simulink model "cddDcmApp"  (R2022b) (continued)
== system cddDcmApp == (continued)
  VentnActr03ElecErr_VentnActr03ElecErr : Inport  Port=275  OutDataTypeStr=Enum: VentnActr01ElecErr  PortDimensions=1
  VentnActr03OvrTempErr_VentnActr03OvrTempErr : Inport  Port=276  OutDataTypeStr=Enum: VentnActr01OvrTempErr  PortDimensions=1
  VentnActr03VoltErr_VentnActr03VoltErr : Inport  Port=277  OutDataTypeStr=Enum: VentnActr01VoltErr  PortDimensions=1
  VentnActr04ElecErr_VentnActr04ElecErr : Inport  Port=278  OutDataTypeStr=Enum: VentnActr01ElecErr  PortDimensions=1
  VentnActr04OvrTempErr_VentnActr04OvrTempErr : Inport  Port=279  OutDataTypeStr=Enum: VentnActr01OvrTempErr  PortDimensions=1
  VentnActr04VoltErr_VentnActr04VoltErr : Inport  Port=280  OutDataTypeStr=Enum: VentnActr01VoltErr  PortDimensions=1
  VentnActr05ElecErr_VentnActr05ElecErr : Inport  Port=281  OutDataTypeStr=Enum: VentnActr01ElecErr  PortDimensions=1
  VentnActr05OvrTempErr_VentnActr05OvrTempErr : Inport  Port=282  OutDataTypeStr=Enum: VentnActr01OvrTempErr  PortDimensions=1
  VentnActr05VoltErr_VentnActr05VoltErr : Inport  Port=283  OutDataTypeStr=Enum: VentnActr01VoltErr  PortDimensions=1
  VentnActr06ElecErr_VentnActr06ElecErr : Inport  Port=284  OutDataTypeStr=Enum: VentnActr01ElecErr  PortDimensions=1
  VentnActr06OvrTempErr_VentnActr06OvrTempErr : Inport  Port=285  OutDataTypeStr=Enum: VentnActr01OvrTempErr  PortDimensions=1
  VentnActr06VoltErr_VentnActr06VoltErr : Inport  Port=286  OutDataTypeStr=Enum: VentnActr01VoltErr  PortDimensions=1
  VentnActr07ElecErr_VentnActr07ElecErr : Inport  Port=287  OutDataTypeStr=Enum: VentnActr01ElecErr  PortDimensions=1
  VentnActr07OvrTempErr_VentnActr07OvrTempErr : Inport  Port=288  OutDataTypeStr=Enum: VentnActr01OvrTempErr  PortDimensions=1
  VentnActr07VoltErr_VentnActr07VoltErr : Inport  Port=289  OutDataTypeStr=Enum: VentnActr01VoltErr  PortDimensions=1
  VentnActr08ElecErr_VentnActr08ElecErr : Inport  Port=290  OutDataTypeStr=Enum: VentnActr01ElecErr  PortDimensions=1
  VentnActr08OvrTempErr_VentnActr08OvrTempErr : Inport  Port=291  OutDataTypeStr=Enum: VentnActr01OvrTempErr  PortDimensions=1
  VentnActr08VoltErr_VentnActr08VoltErr : Inport  Port=292  OutDataTypeStr=Enum: VentnActr01VoltErr  PortDimensions=1
  VentnActr09ElecErr_VentnActr09ElecErr : Inport  Port=293  OutDataTypeStr=Enum: VentnActr01ElecErr  PortDimensions=1
  VentnActr09OvrTempErr_VentnActr09OvrTempErr : Inport  Port=294  OutDataTypeStr=Enum: VentnActr01OvrTempErr  PortDimensions=1
  VentnActr09VoltErr_VentnActr09VoltErr : Inport  Port=295  OutDataTypeStr=Enum: VentnActr01VoltErr  PortDimensions=1
  BswLifeCycle_LifeCycle : Inport  Port=296  OutDataTypeStr=Enum: CCMLifeCycleModeGroup  PortDimensions=1
  DataServices_DID_4756_DID_4756_Front_Mosfet_Blower_FB : Subsystem
  DataServices_DID_47F3_DID_47F3_AIU_Failure_Reasons_and : Subsystem
  DataServices_DID_47F4_DID_47F4_AQS_Air_Quality : Subsystem
  DataServices_DID_47F5_DID_47F5_TestRunCriteria_TRC : Subsystem
  DataServices_DID_47F6_DID_47F6_CCM_Lin1_Frame : Subsystem
  DataServices_DID_47F7_DID_47F7_CCM_Lin2_Frame : Subsystem
  DataServices_DID_47F8_DID_47F8_CCM_Lin3_Frame : Subsystem
  DataServices_DID_47F9_DID_47F9_Blower_Max_Level_Voltage : Subsystem
  DataServices_DID_4AF7_DID_4AF7_1_DC_Motor_Drive_Request_Number : Subsystem
  DataServices_DID_4AF7_DID_4AF7_2_DC_Motor_Drive_Request_Time : Subsystem
  DataServices_DID_4AF7_DID_4AF7_3_DC_Motor_Drive_Request_Direction : Subsystem
  DataServices_DID_9800_DID_9800_1_Evaporator_Temperature : Subsystem
  DataServices_DID_9800_DID_9800_2_incar_temperature : Subsystem
  DataServices_DID_9800_DID_9800_3_outside_temperature : Subsystem
  DataServices_DID_9800_DID_9800_4_Hex_sensor : Subsystem
  DataServices_DID_9801_DID_9801_1_Front_left_vent_temperature : Subsystem
  DataServices_DID_9801_DID_9801_2_Front_left_floor_temperature : Subsystem
  DataServices_DID_9801_DID_9801_3_Front_right_vent_temperature : Subsystem
  DataServices_DID_9801_DID_9801_4_Front_right_floor_temperature : Subsystem
  DataServices_DID_9802_1_Second_row_left_vent_temperatureSecond_row_vent_temperature : Subsystem
  DataServices_DID_9802_2_Second_row_left_floor_temperatureSecond_row_floor_temperature : Subsystem
  DataServices_DID_9802_DID_9802_3_Second_row_right_vent_temperature : Subsystem
  DataServices_DID_9802_DID_9802_4_Second_row_right_floor_temperature : Subsystem
  DataServices_DID_9803_DID_9803_1_Left_sun_intensity : Subsystem
  DataServices_DID_9803_DID_9803_2_Right_sun_intensity : Subsystem
  DataServices_DID_9803_DID_9803_3_engine_coolant_temperature : Subsystem
  DataServices_DID_9803_DID_9803_4_dew_temperature : Subsystem
  DataServices_DID_9804_DID_9804_1_Relative_Humidity : Subsystem
  DataServices_DID_9804_DID_9804_2_AQS_sensor : Subsystem
  DataServices_DID_9804_DID_9804_3_PM25PM25_in_sensor_concentration : Subsystem
  DataServices_DID_9804_DID_9804_4_PM25_out_sensor_concentration : Subsystem
  DataServices_DID_9805_DID_9805_1_CO2_sensor_concentration : Subsystem
  DataServices_DID_9805_DID_9805_2_Front_windscreen_temperature : Subsystem
  DataServices_DID_9805_DID_9805_3_Rear_cabin_temperature : Subsystem
  DataServices_DID_9805_DID_9805_4_Third_row_air_temperature : Subsystem
  DataServices_DID_9808_DID_9808_RHFA_Actuator_Position : Subsystem
  DataServices_DID_9809_3_actuator_positionSecond_temperature_actuator_position : Subsystem
  DataServices_DID_9809_DID_9809_1_Front_left_temperature_actuator_position : Subsystem
  DataServices_DID_9809_DID_9809_2_Front_right_temperature_actuator_position : Subsystem
  DataServices_DID_9809_DID_9809_4_Second_right_temperature_actuator_position : Subsystem
  DataServices_DID_980A_2_Front_ventfloor_actuator_positionfront_vent_actuator_position : Subsystem
  DataServices_DID_980A_DID_980A_1_Deforst_actuator_position : Subsystem
  DataServices_DID_980A_DID_980A_3_Front_floor_actuator_positionposition : Subsystem
  DataServices_DID_980A_DID_980A_4_Second_row_ventventfloor_actuator_position : Subsystem
  DataServices_DID_980B_DID_980B_10_VGA7Passenger_Right_X_actuator_positon : Subsystem
  DataServices_DID_980B_DID_980B_11_VGA8Passenger_Right_Y_actuator_positon : Subsystem
  DataServices_DID_980B_DID_980B_1_second_row_floor_actuator_position : Subsystem
  DataServices_DID_980B_DID_980B_2_Rec_actuator_position : Subsystem
  DataServices_DID_980B_DID_980B_3_Osa_actuator_position : Subsystem
  DataServices_DID_980B_DID_980B_4_VGA1Driver_Left_X_actuator_positon : Subsystem
  DataServices_DID_980B_DID_980B_5_VGA2Driver_Left_Y_actuator_positon : Subsystem
  DataServices_DID_980B_DID_980B_6_VGA3Driver_Right_X_actuator_positon : Subsystem
  DataServices_DID_980B_DID_980B_7_VGA4Driver_Right_Y_actuator_positon : Subsystem
  DataServices_DID_980B_DID_980B_8_VGA5Passenger_Left_X_actuator_positon : Subsystem
  DataServices_DID_980B_DID_980B_9_VGA6Passenger_Left_Y_actuator_positon : Subsystem
  DataServices_DID_980E_DID_980E_1_Front_Blower_pwm : Subsystem
  DataServices_DID_980E_DID_980E_2_Front_Blower_speedLIN1 : Subsystem
  DataServices_DID_980E_DID_980E_3_Incar_motorActvCmd : Subsystem
  DataServices_DID_980E_DID_980E_4_Rear_blower_speed : Subsystem
  DataServices_DID_980F_Data_Record : Subsystem
  DataServices_DID_987B_DID_987B_HBMF_Failure : Subsystem
  DataServices_DID_987C_DID_987C_HBMF_Feedback : Subsystem
  DataServices_DID_987D_DID_987D_PMSI_Failure : Subsystem
  DataServices_DID_987E_DID_987E_AFU_Failure : Subsystem
  DataServices_DID_987F_DID_987F_CCM_Low_Voltage_Power_Limited : Subsystem
  DataServices_DID_9880_DID_9880_PWM_Blower_Error_Internal_Info : Subsystem
  DataServices_DID_988E_DID_988E_Front_PWM_Blower_Feedback : Subsystem
  DataServices_DID_988F_DID_988F_Rear_Blower_Feedback : Subsystem
  DataServices_DID_9890_DID_9890_VGA1_Failure : Subsystem
  DataServices_DID_9891_DID_9891_VGA2_Failure : Subsystem
  DataServices_DID_9892_DID_9892_VGA3_Failure : Subsystem
  DataServices_DID_9893_DID_9893_VGA4_Failure : Subsystem
  DataServices_DID_9894_DID_9894_VGA5_Failure : Subsystem
  DataServices_DID_9895_DID_9895_VGA6_Failure : Subsystem
  DataServices_DID_9896_DID_9896_VGA7_Failure : Subsystem
  DataServices_DID_9897_DID_9897_VGA8_Failure : Subsystem
  DataServices_DID_9898_DID_9898_Sensor_AD : Subsystem
  DataServices_DID_989C_DID_989C_Rear_LIN_Blower_Module_VBMR_Fan_Status : Subsystem
  DataServices_DID_989D_DID_989D_Rear_LIN_Blower_Module_VBMR_Feedback_Datalog : Subsystem
  DataServices_DID_989E_DID_989E_Rear_HVAC_Flap_Actuator_RHFA_Failure_Reasons : Subsystem
  DataServices_DID_989F_DID_989F_CCM_LIN_Actuator_Frame_Status : Subsystem
  DataServices_DID_98A1_DID_98A1_98A1_DC_Motor_Feedback_Voltage : Subsystem
  DataServices_DID_98A2_DID_98A2_Front_Lpm_Blower_Feedback_Voltage_Difference : Subsystem
  DataServices_DID_98A3_DID_98A3_Advanced_Air_Cleaner_AAC_Feedback_Info : Subsystem
  DataServices_DID_98A4_DID_98A4_Oxygen_Generator_Module_OGM_Feedback_Info : Subsystem
  DataServices_DID_98A5_DID_98A5_Interior_Oxygen_Concentration_Sensor_IOSM_Module_Feedback_Info : Subsystem
  DataServices_DID_D910_DID_D910_BLW_THM_Output : Subsystem
  DataServices_DID_D922_DID_D922_1_HVAC_Front_Blower_control_level : Subsystem
  DataServices_DID_D922_DID_D922_2_HVAC_Rear_Blower_control_level : Subsystem
  DataServices_DID_D924_DID_D924_1_No_Of_Movements : Subsystem
  DataServices_DID_D924_DID_D924_2_ADC_value_Lower_Endstop_at_0 : Subsystem
  DataServices_DID_D924_DID_D924_3_ADC_value_Upper_Endstop_at_100 : Subsystem
  DataServices_DID_D925_ComplexStructure_2 : Subsystem
  DataServices_DID_D925_DID_D925_1_Requested_Position : Subsystem
  DataServices_DID_D925_DID_D925_2_Actual_Position : Subsystem
  DataServices_DID_D926_DID_D926_1_No_Of_Movements : Subsystem
  DataServices_DID_D926_DID_D926_2_ADC_value_Lower_Endstop_at_0 : Subsystem
  DataServices_DID_D926_DID_D926_3_ADC_value_Upper_Endstop_at_100 : Subsystem
  DataServices_DID_D927_ComplexStructure_2 : Subsystem
  DataServices_DID_D927_DID_D927_1_Requested_Position : Subsystem
  DataServices_DID_D927_DID_D927_2_Actual_Position : Subsystem
  DataServices_DID_D928_DID_D928_1_No_Of_Movements : Subsystem
  DataServices_DID_D928_DID_D928_2_ADC_value_Lower_Endstop_at_0 : Subsystem
  DataServices_DID_D928_DID_D928_3_ADC_value_Upper_Endstop_at_100 : Subsystem
  DataServices_DID_D929_ComplexStructure_2 : Subsystem
  DataServices_DID_D929_DID_D929_1_Requested_Position : Subsystem
  DataServices_DID_D929_DID_D929_2_Actual_Position : Subsystem
  DataServices_DID_D92A_DID_D92A_1_No_Of_Movements : Subsystem
  DataServices_DID_D92A_DID_D92A_2_ADC_value_Lower_Endstop_at_0 : Subsystem
  DataServices_DID_D92A_DID_D92A_3_ADC_value_Upper_Endstop_at_100 : Subsystem
  DataServices_DID_D92B_ComplexStructure_2 : Subsystem
  DataServices_DID_D92B_DID_D92B_1_Requested_Position : Subsystem
  DataServices_DID_D92B_DID_D92B_2_Actual_Position : Subsystem
  DataServices_DID_D92C_DID_D92C_1_No_Of_Movements : Subsystem
  DataServices_DID_D92C_DID_D92C_2_ADC_value_Lower_Endstop_at_0 : Subsystem
  DataServices_DID_D92C_DID_D92C_3_ADC_value_Upper_Endstop_at_100 : Subsystem
  DataServices_DID_D92D_ComplexStructure_2 : Subsystem
  DataServices_DID_D92D_DID_D92D_1_Requested_Position : Subsystem
  DataServices_DID_D92D_DID_D92D_2_Actual_Position : Subsystem
  DataServices_DID_D92E_DID_D92E_1_No_Of_Movements : Subsystem
  DataServices_DID_D92E_DID_D92E_2_ADC_value_Lower_Endstop_at_0 : Subsystem
  DataServices_DID_D92E_DID_D92E_3_ADC_value_Upper_Endstop_at_100 : Subsystem
  DataServices_DID_D92F_ComplexStructure_2 : Subsystem
  DataServices_DID_D92F_DID_D92F_1_Requested_Position : Subsystem
  DataServices_DID_D92F_DID_D92F_2_Actual_Position : Subsystem
  DataServices_DID_D930_DID_D930_1_No_Of_Movements : Subsystem
  DataServices_DID_D930_DID_D930_2_ADC_value_Lower_Endstop_at_0 : Subsystem
  DataServices_DID_D930_DID_D930_3_ADC_value_Upper_Endstop_at_100 : Subsystem
  DataServices_DID_D931_ComplexStructure_2 : Subsystem
  DataServices_DID_D931_DID_D931_1_Requested_Position : Subsystem
  DataServices_DID_D931_DID_D931_2_Actual_Position : Subsystem
  DataServices_DID_D933_DID_D933_1_step_Range : Subsystem
  DataServices_DID_D933_DID_D933_2_No_Of_Movements : Subsystem
  DataServices_DID_D934_ComplexStructure_2 : Subsystem
  DataServices_DID_D934_DID_D934_1_Requested_Position : Subsystem
  DataServices_DID_D934_DID_D934_2_Actual_Position : Subsystem
  DataServices_DID_D935_DID_D935_1_step_Range : Subsystem
  DataServices_DID_D935_DID_D935_2_No_Of_Movements : Subsystem
  DataServices_DID_D936_ComplexStructure_2 : Subsystem
  DataServices_DID_D936_DID_D936_1_Requested_Position : Subsystem
  DataServices_DID_D936_DID_D936_2_Actual_Position : Subsystem
  DataServices_DID_D937_DID_D937_1_step_Range : Subsystem
  DataServices_DID_D937_DID_D937_2_No_Of_Movements : Subsystem
  DataServices_DID_D938_ComplexStructure_2 : Subsystem
  DataServices_DID_D938_DID_D938_1_Requested_Position : Subsystem
  DataServices_DID_D938_DID_D938_2_Actual_Position : Subsystem
  DataServices_DID_D939_DID_D939_1_step_Range : Subsystem
  DataServices_DID_D939_DID_D939_2_No_Of_Movements : Subsystem
  DataServices_DID_D93A_ComplexStructure_2 : Subsystem
  DataServices_DID_D93A_DID_D93A_1_Requested_Position : Subsystem
  DataServices_DID_D93A_DID_D93A_2_Actual_Position : Subsystem
  DataServices_DID_D93B_DID_D93B_1_step_Range : Subsystem
  DataServices_DID_D93B_DID_D93B_2_No_Of_Movements : Subsystem
  DataServices_DID_D93C_ComplexStructure_2 : Subsystem
  DataServices_DID_D93C_DID_D93C_1_Requested_Position : Subsystem
  DataServices_DID_D93C_DID_D93C_2_Actual_Position : Subsystem
  DataServices_DID_D93D_DID_D93D_1_step_Range : Subsystem
  DataServices_DID_D93D_DID_D93D_2_No_Of_Movements : Subsystem
  DataServices_DID_D93E_ComplexStructure_2 : Subsystem
  DataServices_DID_D93E_DID_D93E_1_Requested_Position : Subsystem
  DataServices_DID_D93E_DID_D93E_2_Actual_Position : Subsystem
  DataServices_DID_D93F_DID_D93F_1_step_Range : Subsystem
  DataServices_DID_D93F_DID_D93F_2_No_Of_Movements : Subsystem
  DataServices_DID_D940_ComplexStructure_2 : Subsystem
  DataServices_DID_D940_DID_D940_1_Requested_Position : Subsystem
  DataServices_DID_D940_DID_D940_2_Actual_Position : Subsystem
  DataServices_DID_D941_DID_D941_1_step_Range : Subsystem
  DataServices_DID_D941_DID_D941_2_No_Of_Movements : Subsystem
  DataServices_DID_D942_ComplexStructure_2 : Subsystem
  DataServices_DID_D942_DID_D942_1_Requested_Position : Subsystem
  DataServices_DID_D942_DID_D942_2_Actual_Position : Subsystem
  DataServices_DID_D943_DID_D943_1_step_Range : Subsystem
  DataServices_DID_D943_DID_D943_2_No_Of_Movements : Subsystem
  DataServices_DID_D944_ComplexStructure_2 : Subsystem
  DataServices_DID_D944_DID_D944_1_Requested_Position : Subsystem
  DataServices_DID_D944_DID_D944_2_Actual_Position : Subsystem
  DataServices_DID_D945_DID_D945_1_step_Range : Subsystem
  DataServices_DID_D945_DID_D945_2_No_Of_Movements : Subsystem
  DataServices_DID_D946_ComplexStructure_2 : Subsystem
  DataServices_DID_D946_DID_D946_1_Requested_Position : Subsystem
  DataServices_DID_D946_DID_D946_2_Actual_Position : Subsystem
  DataServices_DID_D947_DID_D947_1_step_Range : Subsystem
  DataServices_DID_D947_DID_D947_2_No_Of_Movements : Subsystem
  DataServices_DID_D948_ComplexStructure_2 : Subsystem
  DataServices_DID_D948_DID_D948_1_Requested_Position : Subsystem
  DataServices_DID_D948_DID_D948_2_Actual_Position : Subsystem
  DataServices_DID_D94C_THM_SW_Version_DID_D94C_1_THM_SW_Version : Subsystem
  DataServices_DID_D94C_THM_SW_Version_DID_D94C_2_Calibration_Version : Subsystem
  DataServices_DID_D950_DID_D950_1_DC_motor_Maximum_Calibration_limit : Subsystem
  DataServices_DID_D950_DID_D950_2_DC_motor_Actual_ignition_counter : Subsystem
  DataServices_DID_D950_DID_D950_3_HFA_step_motor_Maximum_Calibration_limit : Subsystem
  DataServices_DID_D950_DID_D950_4_HFA_step_motor_Actual_ignition_counter : Subsystem
  DataServices_DID_D950_DID_D950_5_VGA_step_Maximum_Calibration_limit : Subsystem
  DataServices_DID_D950_DID_D950_6_VGA_step_Actual_ignition_counter : Subsystem
  DataServices_DID_D951_DID_D951_1_AQS_HS_Smart_Control : Subsystem
  DataServices_DID_D951_DID_D951_2_VBAT_short_circuit_detection : Subsystem
  DataServices_DID_D951_DID_D951_3_Rear_ETXV_LS_Smart_Control : Subsystem
  DataServices_DID_D951_DID_D951_4_LIN_Power_Supply : Subsystem
  DataServices_DID_D951_DID_D951_5_Rear_PTC_LS_Smart_Control : Subsystem
  DataServices_DID_D951_DID_D951_6_VGARHFA_motor_power_switch : Subsystem
  DataServices_DID_D957_DID_D957_MistRiskLvl : Subsystem
  DataServices_DID_D958_DID_D958_1_step_Range : Subsystem
  DataServices_DID_D958_DID_D958_2_No_Of_Movements : Subsystem
  DataServices_DID_D959_ComplexStructure_2 : Subsystem
  DataServices_DID_D959_DID_D959_1_Requested_Position : Subsystem
  DataServices_DID_D959_DID_D959_2_Actual_Position : Subsystem
  DataServices_DID_D95A_DID_D95A_1_step_Range : Subsystem
  DataServices_DID_D95A_DID_D95A_2_No_Of_Movements : Subsystem
  DataServices_DID_D95B_ComplexStructure_2 : Subsystem
  DataServices_DID_D95B_DID_D95B_1_Requested_Position : Subsystem
  DataServices_DID_D95B_DID_D95B_2_Actual_Position : Subsystem
  DataServices_DID_D95C_DID_D95C_1_step_Range : Subsystem
  DataServices_DID_D95C_DID_D95C_2_No_Of_Movements : Subsystem
  DataServices_DID_D95D_ComplexStructure_2 : Subsystem
  DataServices_DID_D95D_DID_D95D_1_Requested_Position : Subsystem
  DataServices_DID_D95D_DID_D95D_2_Actual_Position : Subsystem
  DataServices_DID_D95E_DID_D95E_1_step_Range : Subsystem
  DataServices_DID_D95E_DID_D95E_2_No_Of_Movements : Subsystem
  DataServices_DID_D95F_ComplexStructure_2 : Subsystem
  DataServices_DID_D95F_DID_D95F_1_Requested_Position : Subsystem
  DataServices_DID_D95F_DID_D95F_2_Actual_Position : Subsystem
  DataServices_DID_D960_DID_D960_1_step_Range : Subsystem
  DataServices_DID_D960_DID_D960_2_No_Of_Movements : Subsystem
  DataServices_DID_D961_ComplexStructure_2 : Subsystem
  DataServices_DID_D961_DID_D961_1_Requested_Position : Subsystem
  DataServices_DID_D961_DID_D961_2_Actual_Position : Subsystem
  DataServices_DID_D962_DID_D962_1_step_Range : Subsystem
  DataServices_DID_D962_DID_D962_2_No_Of_Movements : Subsystem
  DataServices_DID_D963_ComplexStructure_2 : Subsystem
  DataServices_DID_D963_DID_D963_1_Requested_Position : Subsystem
  DataServices_DID_D963_DID_D963_2_Actual_Position : Subsystem
  DataServices_DID_D964_DID_D964_1_step_Range : Subsystem
  DataServices_DID_D964_DID_D964_2_No_Of_Movements : Subsystem
  DataServices_DID_D965_ComplexStructure_2 : Subsystem
  DataServices_DID_D965_DID_D965_1_Requested_Position : Subsystem
  DataServices_DID_D965_DID_D965_2_Actual_Position : Subsystem
  DataServices_DID_D966_DID_D966_1_step_Range : Subsystem
  DataServices_DID_D966_DID_D966_2_No_Of_Movements : Subsystem
  DataServices_DID_D967_ComplexStructure_2 : Subsystem
  DataServices_DID_D967_DID_D967_1_Requested_Position : Subsystem
  DataServices_DID_D967_DID_D967_2_Actual_Position : Subsystem
  DataServices_DID_D968_DID_D968_1_step_Range : Subsystem
  DataServices_DID_D968_DID_D968_2_No_Of_Movements : Subsystem
  DataServices_DID_D969_ComplexStructure_2 : Subsystem
  DataServices_DID_D969_DID_D969_1_Requested_Position : Subsystem
  DataServices_DID_D969_DID_D969_2_Actual_Position : Subsystem
  DataServices_DID_D970_Stepper_Motor_12_Mode_Panel_First_Left_Flap_DID_D970_1_step_Range : Subsystem
  DataServices_DID_D970_Stepper_Motor_12_Mode_Panel_First_Left_Flap_DID_D970_2_No_Of_Movements : Subsystem
  DataServices_DID_D971_Stepper_Motor_12_Mode_Panel_First_Left_Flap_position_ComplexStructure_2 : Subsystem
  DataServices_DID_D971_Stepper_Motor_12_Mode_Panel_First_Left_Flap_position_DID_D971_1_Requested_Position : Subsystem
  DataServices_DID_D971_Stepper_Motor_12_Mode_Panel_First_Left_Flap_position_DID_D971_2_Actual_Position : Subsystem
  DataServices_DID_D972_Stepper_Motor_13_Mode_Panel_First_Side_Left_Flap_DID_D972_1_step_Range : Subsystem
  DataServices_DID_D972_Stepper_Motor_13_Mode_Panel_First_Side_Left_Flap_DID_D972_2_No_Of_Movements : Subsystem
  DataServices_DID_D973_Stepper_Motor_13_Mode_Panel_First_Side_Left_Flap_position_ComplexStructure_2 : Subsystem
  DataServices_DID_D973_Stepper_Motor_13_Mode_Panel_First_Side_Left_Flap_position_DID_D973_1_Requested_Position : Subsystem
  DataServices_DID_D973_Stepper_Motor_13_Mode_Panel_First_Side_Left_Flap_position_DID_D973_2_Actual_Position : Subsystem
  DataServices_DID_D974_Stepper_Motor_14_Mode_Panel_First_Right_Flap_DID_D974_1_step_Range : Subsystem
  DataServices_DID_D974_Stepper_Motor_14_Mode_Panel_First_Right_Flap_DID_D974_2_No_Of_Movements : Subsystem
  DataServices_DID_D975_Stepper_Motor_14_Mode_Panel_First_Right_Flap_position_ComplexStructure_2 : Subsystem
  DataServices_DID_D975_Stepper_Motor_14_Mode_Panel_First_Right_Flap_position_DID_D975_1_Requested_Position : Subsystem
  DataServices_DID_D975_Stepper_Motor_14_Mode_Panel_First_Right_Flap_position_DID_D975_2_Actual_Position : Subsystem
  DataServices_DID_D976_Stepper_Motor_15_Mode_Panel_First_Side_Right_Flap_DID_D976_1_step_Range : Subsystem
  DataServices_DID_D976_Stepper_Motor_15_Mode_Panel_First_Side_Right_Flap_DID_D976_2_No_Of_Movements : Subsystem
  DataServices_DID_D977_Stepper_Motor_15_Mode_Panel_First_Side_Right_Flap_position_ComplexStructure_2 : Subsystem
  DataServices_DID_D977_Stepper_Motor_15_Mode_Panel_First_Side_Right_Flap_position_DID_D977_1_Requested_Position : Subsystem
  DataServices_DID_D977_Stepper_Motor_15_Mode_Panel_First_Side_Right_Flap_position_DID_D977_2_Actual_Position : Subsystem
  DataServices_DID_D978_Stepper_Motor_16_Mode_Floor_First_Left_Flap_DID_D978_1_step_Range : Subsystem
  DataServices_DID_D978_Stepper_Motor_16_Mode_Floor_First_Left_Flap_DID_D978_2_No_Of_Movements : Subsystem
  DataServices_DID_D979_Stepper_Motor_16_Mode_Floor_First_Left_Flap_position_ComplexStructure_2 : Subsystem
  DataServices_DID_D979_Stepper_Motor_16_Mode_Floor_First_Left_Flap_position_DID_D979_1_Requested_Position : Subsystem
  DataServices_DID_D979_Stepper_Motor_16_Mode_Floor_First_Left_Flap_position_DID_D979_2_Actual_Position : Subsystem
  DataServices_DID_D97A_Stepper_Motor_17_Mode_Floor_First_Right_Flap_DID_D97A_1_step_Range : Subsystem
  DataServices_DID_D97A_Stepper_Motor_17_Mode_Floor_First_Right_Flap_DID_D97A_2_No_Of_Movements : Subsystem
  DataServices_DID_D97B_Stepper_Motor_17_Mode_Floor_First_Right_Flap_position_ComplexStructure_2 : Subsystem
  DataServices_DID_D97B_Stepper_Motor_17_Mode_Floor_First_Right_Flap_position_DID_D97B_1_Requested_Position : Subsystem
  DataServices_DID_D97B_Stepper_Motor_17_Mode_Floor_First_Right_Flap_position_DID_D97B_2_Actual_Position : Subsystem
  DataServices_DID_D97C_Stepper_Motor_18_Mode_PanelFloor_Second_Left_Flap_DID_D97C_1_step_Range : Subsystem
  DataServices_DID_D97C_Stepper_Motor_18_Mode_PanelFloor_Second_Left_Flap_DID_D97C_2_No_Of_Movements : Subsystem
  DataServices_DID_D97D_Stepper_Motor_18_Mode_PanelFloor_Second_Left_Flap_position_ComplexStructure_2 : Subsystem
  DataServices_DID_D97D_Stepper_Motor_18_Mode_PanelFloor_Second_Left_Flap_position_DID_D97D_1_Requested_Position : Subsystem
  DataServices_DID_D97D_Stepper_Motor_18_Mode_PanelFloor_Second_Left_Flap_position_DID_D97D_2_Actual_Position : Subsystem
  DataServices_DID_D97E_Stepper_Motor_19_Mode_PanelFloor_Second_Right_Flap_DID_D97E_1_step_Range : Subsystem
  DataServices_DID_D97E_Stepper_Motor_19_Mode_PanelFloor_Second_Right_Flap_DID_D97E_2_No_Of_Movements : Subsystem
  DataServices_DID_D97F_Stepper_Motor_19_Mode_PanelFloor_Second_Right_Flap_position_ComplexStructure_2 : Subsystem
  DataServices_DID_D97F_Stepper_Motor_19_Mode_PanelFloor_Second_Right_Flap_position_DID_D97F_1_Requested_Position : Subsystem
  DataServices_DID_D97F_Stepper_Motor_19_Mode_PanelFloor_Second_Right_Flap_position_DID_D97F_2_Actual_Position : Subsystem
  DataServices_DID_D980_Vent_Grill_Actuator9_Second_Left_X_Flap_DID_D980 : Subsystem
  DataServices_DID_D980_Vent_Grill_Actuator9_Second_Left_X_Flap_DID_D980_2_No_Of_Movements : Subsystem
  DataServices_DID_D981_Vent_Grill_Actuator9_Second_Left_X_Flap_position_ComplexStructure_2 : Subsystem
  DataServices_DID_D981_Vent_Grill_Actuator9_Second_Left_X_Flap_position_DID_D981_1_Requested_Position : Subsystem
  DataServices_DID_D981_Vent_Grill_Actuator9_Second_Left_X_Flap_position_DID_D981_2_Actual_Position : Subsystem
  DataServices_DID_D982_Vent_Grill_Actuator10_Second_Left_Y_Flap_DID_D982_1_step_Range : Subsystem
  DataServices_DID_D982_Vent_Grill_Actuator10_Second_Left_Y_Flap_DID_D982_2_No_Of_Movements : Subsystem
  DataServices_DID_D983_Vent_Grill_Actuator10_Second_Left_Y_Flap_position_ComplexStructure_2 : Subsystem
  DataServices_DID_D983_Vent_Grill_Actuator10_Second_Left_Y_Flap_position_DID_D983_1_Requested_Position : Subsystem
  DataServices_DID_D983_Vent_Grill_Actuator10_Second_Left_Y_Flap_position_DID_D983_2_Actual_Position : Subsystem
  DataServices_DID_D984_Vent_Grill_Actuator11_Second_Right_X_Flap_DID_D984_1_step_Range : Subsystem
  DataServices_DID_D984_Vent_Grill_Actuator11_Second_Right_X_Flap_DID_D984_2_No_Of_Movements : Subsystem
  DataServices_DID_D985_Vent_Grill_Actuator11_Second_Right_X_Flap_position_ComplexStructure_2 : Subsystem
  DataServices_DID_D985_Vent_Grill_Actuator11_Second_Right_X_Flap_position_DID_D985_1_Requested_Position : Subsystem
  DataServices_DID_D985_Vent_Grill_Actuator11_Second_Right_X_Flap_position_DID_D985_2_Actual_Position : Subsystem
  DataServices_DID_D986_Vent_Grill_Actuator12_Second_Right_Y_Flap_DID_D986_1_step_Range : Subsystem
  DataServices_DID_D986_Vent_Grill_Actuator12_Second_Right_Y_Flap_DID_D986_2_No_Of_Movements : Subsystem
  DataServices_DID_D987_Vent_Grill_Actuator12_Second_Right_Y_Flap_position_ComplexStructure_2 : Subsystem
  DataServices_DID_D987_Vent_Grill_Actuator12_Second_Right_Y_Flap_position_DID_D987_1_Requested_Position : Subsystem
  DataServices_DID_D987_Vent_Grill_Actuator12_Second_Right_Y_Flap_position_DID_D987_2_Actual_Position : Subsystem
  DataServices_DID_D988_Vent_Grill_Actuator13_Second_Right_Y_Flap_DID_D988_1_step_Range : Subsystem
  DataServices_DID_D988_Vent_Grill_Actuator13_Second_Right_Y_Flap_DID_D988_2_No_Of_Movements : Subsystem
  DataServices_DID_D989_Vent_Grill_Actuator13_Second_Right_Y_Flap_position_DID_D989_1_Requested_Position : Subsystem
  DataServices_DID_D989_Vent_Grill_Actuator13_Second_Right_Y_Flap_position_DID_D989_2_Actual_Position : Subsystem
  DataServices_DID_D989_Vent_Grill_Actuator13_Second_Right_Y_Flap_position_DID_D989_3_Calibration_status : Subsystem
  DataServices_DID_D989_Vent_Grill_Actuator13_Second_Right_Y_Flap_position_DID_D989_4_Working_Status : Subsystem
  DataServices_DID_D98A_Vent_Grill_Actuator14_Second_Right_Y_Flap_DID_D98A_1_step_Range : Subsystem
  DataServices_DID_D98A_Vent_Grill_Actuator14_Second_Right_Y_Flap_DID_D98A_2_No_Of_Movements : Subsystem
  DataServices_DID_D98B_Vent_Grill_Actuator14_Second_Right_Y_Flap_position_DID_D98B_1_Requested_Position : Subsystem
  DataServices_DID_D98B_Vent_Grill_Actuator14_Second_Right_Y_Flap_position_DID_D98B_2_Actual_Position : Subsystem
  DataServices_DID_D98B_Vent_Grill_Actuator14_Second_Right_Y_Flap_position_DID_D98B_3_Calibration_Status : Subsystem
  DataServices_DID_D98B_Vent_Grill_Actuator14_Second_Right_Y_Flap_position_DID_D98B_4_Working_Status : Subsystem
  DataServices_DID_D98C_Vent_Grill_Actuator15_Second_Right_Y_Flap_DID_D98C_1_step_Range : Subsystem
  DataServices_DID_D98C_Vent_Grill_Actuator15_Second_Right_Y_Flap_DID_D98C_2_No_Of_Movements : Subsystem
  DataServices_DID_D98D_Vent_Grill_Actuator15_Second_Right_Y_Flap_position_ComplexStructure_2 : Subsystem
  DataServices_DID_D98D_Vent_Grill_Actuator15_Second_Right_Y_Flap_position_DID_D98D_1_Requested_Position : Subsystem
  DataServices_DID_D98D_Vent_Grill_Actuator15_Second_Right_Y_Flap_position_DID_D98D_2_Actual_Position : Subsystem
  DataServices_DID_D98E_Vent_Grill_Actuator16_Second_Right_Y_Flap_DID_D98E_1_step_Range : Subsystem
  DataServices_DID_D98E_Vent_Grill_Actuator16_Second_Right_Y_Flap_DID_D98E_2_No_Of_Movements : Subsystem
  DataServices_DID_D98F_Vent_Grill_Actuator16_Second_Right_Y_Flap_position_ComplexStructure_2 : Subsystem
  DataServices_DID_D98F_Vent_Grill_Actuator16_Second_Right_Y_Flap_position_DID_D98F_1_Requested_Position : Subsystem
  DataServices_DID_D98F_Vent_Grill_Actuator16_Second_Right_Y_Flap_position_DID_D98F_2_Actual_Position : Subsystem
  DataServices_DID_DA00_DID_DA00_Read_CCM_Internal_Data_For : Subsystem
  Dcm_Reset_Hard_sys : Subsystem
  Dcm_Reset_JumpToBootLoader_sys : Subsystem
  DiagSessionCtrl_Default_sys : Subsystem
  RoutineServices_RID_DF20_Run_Calibration_on_All_Damper_Motors : Subsystem
  RoutineServices_RID_DF40_HFA_motor_auto_addressing : Subsystem
  cddDcmApp_20ms_Runnable_sys : Subsystem
  cddDcmApp_Init_1 : Subsystem
  cddDcmApp_Init_sys : Subsystem
  wires:
  AACAirClngErrStsNgcc_AACAirClngErrStsNgcc/1 -> cddDcmApp_20ms_Runnable_sys/1(AACAirClngErrStsNgcc_AACAirClngErrStsNgcc_read)
  AACAirClngStsNgcc_AACAirClngStsNgcc/1 -> cddDcmApp_20ms_Runnable_sys/2(AACAirClngStsNgcc_AACAirClngStsNgcc_read)
  AfuFrameSts_AfuFrameSts/1 -> cddDcmApp_20ms_Runnable_sys/3(AfuFrameSts_AfuFrameSts_read)
  AFUGenErr_AFUGenErr/1 -> cddDcmApp_20ms_Runnable_sys/4(AFUGenErr_AFUGenErr_read)
  AirClngErrSts_AirClngErrSts/1 -> cddDcmApp_20ms_Runnable_sys/5(AirClngErrSts_AirClngErrSts_read)
  AirClngSts_AirClngSts/1 -> cddDcmApp_20ms_Runnable_sys/6(AirClngSts_AirClngSts_read)
  AirFragFanErr_AirFragFanErr/1 -> cddDcmApp_20ms_Runnable_sys/7(AirFragFanErr_AirFragFanErr_read)
  AirFragMot1Err_AirFragMot1Err/1 -> cddDcmApp_20ms_Runnable_sys/8(AirFragMot1Err_AirFragMot1Err_read)
  AirFragMot2Err_AirFragMot2Err/1 -> cddDcmApp_20ms_Runnable_sys/9(AirFragMot2Err_AirFragMot2Err_read)
  AirFragMot3Err_AirFragMot3Err/1 -> cddDcmApp_20ms_Runnable_sys/10(AirFragMot3Err_AirFragMot3Err_read)
  AirFragMot4Err_AirFragMot4Err/1 -> cddDcmApp_20ms_Runnable_sys/11(AirFragMot4Err_AirFragMot4Err_read)
  AirFragMot5Err_AirFragMot5Err/1 -> cddDcmApp_20ms_Runnable_sys/12(AirFragMot5Err_AirFragMot5Err_read)
  AirFragOvrVoltErr_AirFragOvrVoltErr/1 -> cddDcmApp_20ms_Runnable_sys/13(AirFragOvrVoltErr_AirFragOvrVoltErr_read)
  AirFragTempErr_AirFragTempErr/1 -> cddDcmApp_20ms_Runnable_sys/14(AirFragTempErr_AirFragTempErr_read)
  AirFragUndVoltErr_AirFragUndVoltErr/1 -> cddDcmApp_20ms_Runnable_sys/15(AirFragUndVoltErr_AirFragUndVoltErr_read)
  AirTFromHeatgEstimdFirstLeForCCM_AirTFromHeatgEstimdFirstLeForCCM/1 -> cddDcmApp_20ms_Runnable_sys/16(AirTFromHeatgEstimdFirstLeForCCM_AirTFromHeatgEstimdFirstLeForCCM_read)
  AirTFromHeatgEstimdFirstRiForCCM_AirTFromHeatgEstimdFirstRiForCCM/1 -> cddDcmApp_20ms_Runnable_sys/17(AirTFromHeatgEstimdFirstRiForCCM_AirTFromHeatgEstimdFirstRiForCCM_read)
  AirTFromHeatgEstimdForCCMForRead_AirTFromHeatgEstimdForCCMForRead/1 -> cddDcmApp_20ms_Runnable_sys/18(AirTFromHeatgEstimdForCCMForRead_AirTFromHeatgEstimdForCCMForRead_read)
  AirTFromHeatgEstimdReForCCM_AirTFromHeatgEstimdReForCCM/1 -> cddDcmApp_20ms_Runnable_sys/19(AirTFromHeatgEstimdReForCCM_AirTFromHeatgEstimdReForCCM_read)
  AirTFromHeatgEstimdSecLeForCCM_AirTFromHeatgEstimdSecLeForCCM/1 -> cddDcmApp_20ms_Runnable_sys/20(AirTFromHeatgEstimdSecLeForCCM_AirTFromHeatgEstimdSecLeForCCM_read)
  AiuFrameSts_AiuFrameSts/1 -> cddDcmApp_20ms_Runnable_sys/21(AiuFrameSts_AiuFrameSts_read)
  AmbTFildForClimaNgcc_AmbTFildForClima/1 -> cddDcmApp_20ms_Runnable_sys/22(AmbTFildForClimaNgcc_AmbTFildForClima_read)
  AmbTRaw_AmbTRaw/1 -> cddDcmApp_20ms_Runnable_sys/23(AmbTRaw_AmbTRaw_read)
  AQSAirQuality_AQSAirQuality/1 -> cddDcmApp_20ms_Runnable_sys/24(AQSAirQuality_AQSAirQuality_read)
  AQSCOxstatus_AQSCOxstatus/1 -> cddDcmApp_20ms_Runnable_sys/25(AQSCOxstatus_AQSCOxstatus_read)
  AqsFrameSts_AqsFrameSts/1 -> cddDcmApp_20ms_Runnable_sys/26(AqsFrameSts_AqsFrameSts_read)
  AQSNH3status_AQSNH3status/1 -> cddDcmApp_20ms_Runnable_sys/27(AQSNH3status_AQSNH3status_read)
  AQSNoxStatus_AQSNoxStatus/1 -> cddDcmApp_20ms_Runnable_sys/28(AQSNoxStatus_AQSNoxStatus_read)
  BkpOfDstTrvld_BkpOfDstTrvld/1 -> cddDcmApp_20ms_Runnable_sys/29(BkpOfDstTrvld_BkpOfDstTrvld_read)
  BkpOfDstTrvld_BkpOfDstTrvld_IsUpdated/1 -> cddDcmApp_20ms_Runnable_sys/30(BkpOfDstTrvld_BkpOfDstTrvld_IsUpdated_read)
  CarCfgForClimaCtrl_CarCfgForAirClng/1 -> cddDcmApp_20ms_Runnable_sys/31(CarCfgForClimaCtrl_CarCfgForAirClng_read)
  CarCfgForClimaCtrl_CarCfgForAirClnrAdv/1 -> cddDcmApp_20ms_Runnable_sys/32(CarCfgForClimaCtrl_CarCfgForAirClnrAdv_read)
  CarCfgForClimaCtrl_CarCfgForAirFrag/1 -> cddDcmApp_20ms_Runnable_sys/33(CarCfgForClimaCtrl_CarCfgForAirFrag_read)